simulink model "wlan_phy_rx_pmd"  (Simulink 7.8)
settings: Solver=VariableStepDiscrete  SolverMode=SingleTasking  StartTime=0.0  StopTime=rx_sim_time  RelTol=1e-3
PostLoadFcn:
  | wlan_phy_rx_init
InitFcn:
  | wlan_phy_rx_init
StartFcn:
  | wlan_phy_rx_init
values from neighbouring files (symbols this model uses):
  barker_seq20 = 0.95 * circshift(barker_seq20, [0 4]) ./ max(abs(barker_seq20))    [wlan_phy_rx_init.m]
  bit_scrambler_lfsr_bytes = sum(bit_scrambler_lfsr_bytes .* repmat(2.^[0:7], 127, 1),2)    [wlan_phy_rx_init.m]
  deinterleave_ROM = [deinterleave_ROM interleave_11n_64QAM zeros(1, 512-length(interleave_11n_64QAM))]    [wlan_phy_rx_init.m]
  longCorr_coef_i = [3*real(longCorr_coef)]    [wlan_phy_rx_init.m]
  longCorr_coef_q = [3*imag(longCorr_coef)]    [wlan_phy_rx_init.m]
  MAX_CP_LEN = 32    [wlan_phy_rx_init.m]
  MAX_NCBPS = NCBPS_11n_64QAM    (= 312)    [wlan_phy_rx_init.m]
  MAX_NUM_BYTES = 2^16-1    (= 65535)    [wlan_phy_rx_init.m]
  MAX_NUM_SC = 64    [wlan_phy_rx_init.m]
  MAX_NUM_SYMS = 600    [wlan_phy_rx_init.m]
  NCBPS_11a_BPSK = 48    [wlan_phy_rx_init.m]
  NDBPS_11a_BPSK12 = 24    [wlan_phy_rx_init.m]
  PHY_SIGNAL_MIN_LEN = 14    [wlan_phy_rx_init.m]
  preFFT_sampBuff_numSamps = 4*MAX_NUM_SC    (= 256)    [wlan_phy_rx_init.m]
  rx_sim_sig_samp_time = 8    [wlan_phy_rx_init.m]
  scr_ind_rev = zeros(1,128)    [wlan_phy_rx_init.m]
undefined symbols (not defined in the file or in its folder): gcbh, mcs_rom_11ag, mcs_rom_11n, xlBlockLoadCallback, xlGetNormalizedPeriod, xlipgetpartsetting
libraries: xbsIndex_r4 x3862, simulink x1, xbsEDKLib_r4 x1
(part 6 of 23)

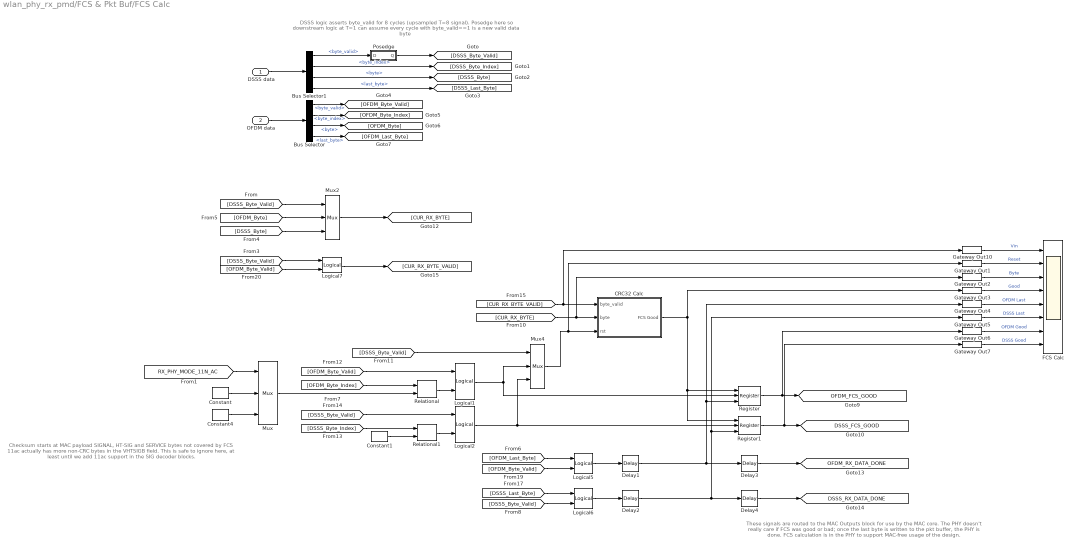
== system wlan_phy_rx_pmd/FCS & Pkt Buf/FCS Calc ==
  note: These signals are routed to the MAC Outputs
      | block for use by the MAC core. The PHY doesn't
      | really care if FCS was good or bad; once the
      | last byte is written to the pkt buffer, the PHY is done.
      | FCS calculation is in the PHY to support MAC-free
      | usage of the design.
  note: DSSS logic asserts byte_valid for 8 cycles (upsampled T=8 signal).
      | Posedge here so downstream logic at T=1 can assume every cycle
      | with byte_valid==1 is a new valid data byte
  note: Checksum starts at MAC payload
      | SIGNAL, HT-SIG and SERVICE
      | bytes not covered by FCS
      |
      | 11ac actually has more non-CRC bytes
      | in the VHTSIGB field. This is safe to
      | ignore here, at least until we add 11ac
      | support in the SIG decoder blocks.
  DSSS data : Inport
  OFDM data : Inport  Port=2
  Bus Selector : BusSelector  OutputSignals=byte_valid,byte_index,byte,last_byte
  Bus Selector1 : BusSelector  OutputSignals=byte_valid,byte_index,byte,last_byte
  CRC32 Calc : Subsystem
  Constant : xbsIndex_r4/Constant  const=4  gui_display_data_type=Fixed-point  arith_type=Unsigned  n_bits=3  bin_pt=0  preci_type=Single  exp_width=8  frac_width=24  explicit_period=off  period=1  equ=P=C  opselect=C  inp2=PCIN>>17  opr=+  inp1=P  carry=CIN  dbl_ovrd=off  block_type=constant
  Constant1 : xbsIndex_r4/Constant  const=5  gui_display_data_type=Fixed-point  arith_type=Unsigned  n_bits=3  bin_pt=0  preci_type=Single  exp_width=8  frac_width=24  explicit_period=off  period=1  equ=P=C  opselect=C  inp2=PCIN>>17  opr=+  inp1=P  carry=CIN  dbl_ovrd=off  block_type=constant
  Constant4 : xbsIndex_r4/Constant  const=10  gui_display_data_type=Fixed-point  arith_type=Unsigned  n_bits=4  bin_pt=0  preci_type=Single  exp_width=8  frac_width=24  explicit_period=off  period=1  equ=P=C  opselect=C  inp2=PCIN>>17  opr=+  inp1=P  carry=CIN  dbl_ovrd=off  block_type=constant
  Delay{1..4} (4 alike) : xbsIndex_r4/Delay  rst=off  infoeditControl=Selection of Reset will increase slice count due to use of real FFs and instead of SRLs  en=off  latency=1  dbl_ovrd=off  reg_retiming=off  block_type=delay
  FCS Calc : Scope  NumInputPorts=8
      settings: TimeRange=16000  YMin=0~0~0~-1~0~-1~-1~-1  YMax=1~1~275~1~1~1~1~1  SaveName=ScopeData22
  Gateway Out{1..7,10} (8 alike) : xbsIndex_r4/Gateway Out  inherit_from_input=off  hdl_port=off  timing_constraint=None  locs_specified=off  LOCs={}  UseAsDAC=off  DACChannel='1'  block_type=gatewayout
  Logical{1..2,5..6} (4 alike) : xbsIndex_r4/Logical  logical_function=AND  inputs=2  en=off  latency=0  precision=Full  arith_type=Unsigned  n_bits=8  bin_pt=2  align_bp=on  dbl_ovrd=off  block_type=logical
      settings: xl_area=[0, 0, 0, 0, 0, 0, 0]  block_version=VER_STRING_GOES_HERE
  Logical7 : xbsIndex_r4/Logical  logical_function=OR  inputs=2  en=off  latency=0  precision=Full  arith_type=Unsigned  n_bits=8  bin_pt=2  align_bp=on  dbl_ovrd=off  block_type=logical
      settings: xl_area=[0, 0, 0, 0, 0, 0, 0]  block_version=VER_STRING_GOES_HERE
  Mux : xbsIndex_r4/Mux  inputs=2  en=off  latency=0  precision=Full  arith_type=Unsigned  n_bits=16  bin_pt=14  quantization=Truncate  overflow=Wrap  dbl_ovrd=off  block_type=mux
  Mux2 : xbsIndex_r4/Mux  inputs=2  en=off  latency=0  precision=Full  arith_type=Unsigned  n_bits=16  bin_pt=14  quantization=Truncate  overflow=Wrap  dbl_ovrd=off  block_type=mux
  Mux4 : xbsIndex_r4/Mux  inputs=2  en=off  latency=0  precision=Full  arith_type=Unsigned  n_bits=16  bin_pt=14  quantization=Truncate  overflow=Wrap  dbl_ovrd=off  block_type=mux
  Posedge : Subsystem
  Register : xbsIndex_r4/Register  init=0  rst=on  en=on  dbl_ovrd=off  block_type=register
  Register1 : xbsIndex_r4/Register  init=0  rst=on  en=on  dbl_ovrd=off  block_type=register
  Relational : xbsIndex_r4/Relational  mode=a<=b  en=off  latency=0  dbl_ovrd=off  block_type=relational
  Relational1 : xbsIndex_r4/Relational  mode=a<=b  en=off  latency=0  dbl_ovrd=off  block_type=relational
  tag scope: DSSS_FCS_GOOD=global, DSSS_RX_DATA_DONE=global, OFDM_FCS_GOOD=global, OFDM_RX_DATA_DONE=global
  wires:
  [DSSS_Byte_Valid] -> Mux2/1
  [OFDM_Byte] -> Mux2/2
  [DSSS_Byte] -> Mux2/3
  Mux2/1 -> [CUR_RX_BYTE]
  [DSSS_Byte_Valid] -> Logical7/1
  [OFDM_Byte_Valid] -> Logical7/2
  Logical7/1 -> [CUR_RX_BYTE_VALID]
  Mux4/1 -> CRC32 Calc/3(rst), Gateway Out1/1
  [DSSS_Byte_Valid] -> Mux4/1
  Register1/1 -> [DSSS_FCS_GOOD], Gateway Out7/1
  Register/1 -> [OFDM_FCS_GOOD], Gateway Out6/1
  CRC32 Calc/1(FCS Good) -> Register/1, Register1/1, Gateway Out3/1
  Delay2/1 -> Delay4/1, Register1/3, Gateway Out5/1
  Delay1/1 -> Delay3/1, Register/3, Gateway Out4/1
  Logical6/1 -> Delay2/1
  Logical5/1 -> Delay1/1
  [DSSS_Byte_Valid] -> Logical6/2
  [DSSS_Last_Byte] -> Logical6/1
  [OFDM_Byte_Valid] -> Logical5/2
  [OFDM_Last_Byte] -> Logical5/1
  [CUR_RX_BYTE_VALID] -> CRC32 Calc/1(byte_valid), Gateway Out10/1
  Logical2/1 -> Mux4/3, Register1/2
  [DSSS_Byte_Valid] -> Logical2/1
  Relational1/1 -> Logical2/2
  Constant1/1 -> Relational1/2
  [DSSS_Byte_Index] -> Relational1/1
  Logical1/1 -> Mux4/2, Register/2
  [OFDM_Byte_Valid] -> Logical1/1
  Relational/1 -> Logical1/2
  Mux/1 -> Relational/2
  [OFDM_Byte_Index] -> Relational/1
  [CUR_RX_BYTE] -> CRC32 Calc/2(byte), Gateway Out2/1
  Delay3/1 -> [OFDM_RX_DATA_DONE]
  Delay4/1 -> [DSSS_RX_DATA_DONE]
  Gateway Out10/1 -> FCS Calc/1  "Vin"
  Gateway Out1/1 -> FCS Calc/2  "Reset"
  Gateway Out2/1 -> FCS Calc/3  "Byte"
  Gateway Out3/1 -> FCS Calc/4  "Good"
  Gateway Out4/1 -> FCS Calc/5  "OFDM Last"
  Gateway Out5/1 -> FCS Calc/6  "DSSS Last"
  Gateway Out6/1 -> FCS Calc/7  "OFDM Good"
  Gateway Out7/1 -> FCS Calc/8  "DSSS Good"
  Bus Selector1/1 -> Posedge/1(D)  "<byte_valid>"
  Bus Selector1/2 -> [DSSS_Byte_Index]  "<byte_index>"
  Bus Selector1/3 -> [DSSS_Byte]  "<byte>"
  Bus Selector1/4 -> [DSSS_Last_Byte]  "<last_byte>"
  Bus Selector/1 -> [OFDM_Byte_Valid]  "<byte_valid>"
  Bus Selector/2 -> [OFDM_Byte_Index]  "<byte_index>"
  Bus Selector/3 -> [OFDM_Byte]  "<byte>"
  Bus Selector/4 -> [OFDM_Last_Byte]  "<last_byte>"
  DSSS data/1 -> Bus Selector1/1
  OFDM data/1 -> Bus Selector/1
  Posedge/1(Q) -> [DSSS_Byte_Valid]
  [RX_PHY_MODE_11N_AC] -> Mux/1
  Constant/1 -> Mux/2
  Constant4/1 -> Mux/3

== system wlan_phy_rx_pmd/FCS & Pkt Buf/Pkt Buf Interface ==
  DSSS data : Inport
  OFDM data : Inport  Port=2
  Addr Check : Subsystem
  BRAM IF 64b : Subsystem
  Bus Selector : BusSelector  OutputSignals=byte_valid,byte_index,byte,last_byte
  Bus Selector1 : BusSelector  OutputSignals=byte_valid,byte_index,byte,last_byte
  DSSS Addr Calc : Subsystem
  Logical7 : xbsIndex_r4/Logical  logical_function=OR  inputs=2  en=off  latency=0  precision=Full  arith_type=Unsigned  n_bits=8  bin_pt=2  align_bp=on  dbl_ovrd=off  block_type=logical
      settings: xl_area=[0, 0, 0, 0, 0, 0, 0]  block_version=VER_STRING_GOES_HERE
  Mux2 : xbsIndex_r4/Mux  inputs=2  en=off  latency=0  precision=Full  arith_type=Unsigned  n_bits=16  bin_pt=14  quantization=Truncate  overflow=Wrap  dbl_ovrd=off  block_type=mux
  Mux3 : xbsIndex_r4/Mux  inputs=2  en=off  latency=0  precision=Full  arith_type=Unsigned  n_bits=16  bin_pt=14  quantization=Truncate  overflow=Wrap  dbl_ovrd=off  block_type=mux
  Mux5 : xbsIndex_r4/Mux  inputs=2  en=off  latency=0  precision=Full  arith_type=Unsigned  n_bits=16  bin_pt=14  quantization=Truncate  overflow=Wrap  dbl_ovrd=off  block_type=mux
  OFDM Addr Calc : Subsystem
  Register{4..5,7..8} (4 alike) : xbsIndex_r4/Register  init=0  rst=off  en=off  dbl_ovrd=off  block_type=register
  Rx Data MAC Outputs : Subsystem
  wires:
  [DSSS_Byte_Valid] -> Mux3/1
  [DSSS_Byte_Index] -> DSSS Addr Calc/1(R)
  DSSS Addr Calc/1(M) -> Mux5/3
  [DSSS_Byte_Valid] -> Mux5/1
  Mux5/1 -> Addr Check/2(Addr)
  [OFDM_Byte_Index] -> OFDM Addr Calc/1(R)
  OFDM Addr Calc/1(M) -> Mux5/2
  Mux3/1 -> Register4/1
  Register4/1 -> BRAM IF 64b/4(Pkt Buf Sel)
  [CUR_RX_BYTE] -> Register7/1
  [CUR_RX_BYTE_VALID] -> Addr Check/1(WrEn)
  Register7/1 -> BRAM IF 64b/1("byte "), Rx Data MAC Outputs/1("Byte ")
  Register8/1 -> BRAM IF 64b/2(byte_valid), Rx Data MAC Outputs/2(Byte Valid)
  Register5/1 -> BRAM IF 64b/3(byte_ind), Rx Data MAC Outputs/3(Byte Ind)
  Bus Selector1/1 -> [DSSS_Byte_Valid]  "<byte_valid>"
  Bus Selector1/2 -> [DSSS_Byte_Index]  "<byte_index>"
  Bus Selector1/3 -> [DSSS_Byte]  "<byte>"
  Bus Selector1/4 -> [DSSS_Last_Byte]  "<last_byte>"
  Bus Selector/1 -> [OFDM_Byte_Valid]  "<byte_valid>"
  Bus Selector/2 -> [OFDM_Byte_Index]  "<byte_index>"
  Bus Selector/3 -> [OFDM_Byte]  "<byte>"
  Bus Selector/4 -> [OFDM_Last_Byte]  "<last_byte>"
  DSSS data/1 -> Bus Selector1/1
  OFDM data/1 -> Bus Selector/1
  [DSSS_Byte_Valid] -> Mux2/1
  [OFDM_Byte] -> Mux2/2
  [DSSS_Byte] -> Mux2/3
  Mux2/1 -> [CUR_RX_BYTE]
  [DSSS_Byte_Valid] -> Logical7/1
  [OFDM_Byte_Valid] -> Logical7/2
  Logical7/1 -> [CUR_RX_BYTE_VALID]
  [regRx_OFDM_PKT_BUF_SEL] -> Mux3/2
  [regRx_DSSS_PKT_BUF_SEL] -> Mux3/3
  Addr Check/1(" WrEn") -> Register8/1
  Addr Check/2(" Addr") -> Register5/1

== system wlan_phy_rx_pmd/FFT/Reset Gen ==
  note: Extend FFT reset for duration of TVALID + 4 to handle
      | case of pkt ending while new FFT frame is being loaded.
      | Turns out reset inputs for FIFO and FFT do not actually
      | flush internal state - both cores keep respnding to inputs
      | while reset is asserted (very frustrating...)
  FFT Valid In : Inport
  Reset : Inport  Port=2
  Inverter : xbsIndex_r4/Inverter  en=off  latency=0  dbl_ovrd=off  block_type=inv
  Inverter1 : xbsIndex_r4/Inverter  en=off  latency=4  dbl_ovrd=off  block_type=inv
  Logical : xbsIndex_r4/Logical  logical_function=AND  inputs=2  en=off  latency=0  precision=Full  arith_type=Unsigned  n_bits=16  bin_pt=0  align_bp=on  dbl_ovrd=off  block_type=logical
  Logical1 : xbsIndex_r4/Logical  logical_function=OR  inputs=2  en=off  latency=0  precision=Full  arith_type=Unsigned  n_bits=16  bin_pt=0  align_bp=on  dbl_ovrd=off  block_type=logical
  Register2 : xbsIndex_r4/Register  init=1  rst=off  en=off  dbl_ovrd=off  block_type=register
      settings: xl_area=[1 1 0 0 0 0 0]  block_version=VER_STRING_GOES_HERE
  S-R Latch1 : Subsystem
  FFT Reset : Outport
  " Reset" : Outport  Port=2
  wires:
  Inverter1/1 -> S-R Latch1/2(R)
  Reset/1 -> Logical1/1, Logical/2
  Logical/1 -> S-R Latch1/1(S)
  S-R Latch1/1(Q) -> Logical1/2
  Logical1/1 -> " Reset"/1, Inverter/1
  FFT Valid In/1 -> Inverter1/1, Logical/1
  Inverter/1 -> Register2/1
  Register2/1 -> FFT Reset/1

== system wlan_phy_rx_pmd/FFT/Sym Count ==
  note: "Symbol index" counts OFDM symbols and increments with each FFT.
      | Content of each OFDM sybmol depends on PHY mode (ag, n, or ac)
  data_in_tvalid : Inport
  pkt_done : Inport  Port=2
  data_out_tlast : Inport  Port=3
  Logical : xbsIndex_r4/Logical  logical_function=AND  inputs=2  en=off  latency=0  precision=Full  arith_type=Unsigned  n_bits=16  bin_pt=0  align_bp=on  dbl_ovrd=off  block_type=logical
  S-R Latch1 : Subsystem
  Symbol Counter : xbsIndex_r4/Counter  cnt_type=Free Running  cnt_to=Inf  operation=Up  start_count=0  cnt_by_val=1  arith_type=Unsigned  n_bits=ceil(log2(MAX_NUM_SYMS))  bin_pt=0  load_pin=off  rst=on  en=on  explicit_period=off  period=1  dbl_ovrd=off  use_behavioral_HDL=off  implementation=Fabric  block_type=counter
  Sym Ind : Outport
  wires:
  data_out_tlast/1 -> Logical/1
  Logical/1 -> Symbol Counter/2
  S-R Latch1/1(Q) -> Logical/2
  data_in_tvalid/1 -> S-R Latch1/1(S)
  Symbol Counter/1 -> Sym Ind/1
  pkt_done/1 -> S-R Latch1/2(R), Symbol Counter/1

== system wlan_phy_rx_pmd/Inputs/RF A Inputs ==
  Rst : Inport
  IQ Valid : Inport  Port=2
  Constant1 : Constant  Value=0
  Constant2 : Constant  Value=2
  Constant4 : Constant  Value=5
  Delay1 : xbsIndex_r4/Delay  rst=off  infoeditControl=Selection of Reset will increase slice count due to use of real FFs and instead of SRLs  en=off  latency=1  dbl_ovrd=off  reg_retiming=off  block_type=delay
  Delay2 : xbsIndex_r4/Delay  rst=off  infoeditControl=Selection of Reset will increase slice count due to use of real FFs and instead of SRLs  en=off  latency=1  dbl_ovrd=off  reg_retiming=off  block_type=delay
  Down Sample : xbsIndex_r4/Down Sample  sample_ratio=16  sample_phase="Last Value of Frame  (most efficient)"  en=off  latency=1  dbl_ovrd=off  block_type=dsamp
  From Workspace : FromWorkspace  VariableName=rx_sim_sig_adc_I  SampleTime=rx_sim_sig_samp_time  Interpolate=off  OutputAfterFinalValue=Setting to zero
  From Workspace1 : FromWorkspace  VariableName=rx_sim_sig_adc_Q  SampleTime=rx_sim_sig_samp_time  Interpolate=off  OutputAfterFinalValue=Setting to zero
  RF A RSSI Sum : Subsystem
  RFA_G_BB : xbsIndex_r4/Gateway In  gui_display_data_type=Fixed-point  arith_type=Unsigned  n_bits=5  bin_pt=0  preci_type=Single  exp_width=8  frac_width=24  quantization="Round  (unbiased: +/- Inf)"  overflow=Saturate  period=1  dbl_ovrd=off  timing_constraint=None  locs_specified=off  LOCs={}  UseAsADC=off  ADCChannel='1'  inherit_from_input=off  hdl_port=on  block_type=gatewayin
  RFA_G_RF : xbsIndex_r4/Gateway In  gui_display_data_type=Fixed-point  arith_type=Unsigned  n_bits=2  bin_pt=0  preci_type=Single  exp_width=8  frac_width=24  quantization="Round  (unbiased: +/- Inf)"  overflow=Saturate  period=1  dbl_ovrd=off  timing_constraint=None  locs_specified=off  LOCs={}  UseAsADC=off  ADCChannel='1'  inherit_from_input=off  hdl_port=on  block_type=gatewayin
  RFA_RSSI : xbsIndex_r4/Gateway In  gui_display_data_type=Fixed-point  arith_type=Unsigned  n_bits=10  bin_pt=0  preci_type=Single  exp_width=8  frac_width=24  quantization="Round  (unbiased: +/- Inf)"  overflow=Saturate  period=1  dbl_ovrd=off  timing_constraint=None  locs_specified=off  LOCs={}  UseAsADC=off  ADCChannel='1'  inherit_from_input=off  hdl_port=on  block_type=gatewayin
  RFA_RX_I : xbsIndex_r4/Gateway In  gui_display_data_type=Fixed-point  arith_type="Signed  (2's comp)"  n_bits=16  bin_pt=15  preci_type=Single  exp_width=8  frac_width=24  quantization="Round  (unbiased: +/- Inf)"  overflow=Saturate  period=1  dbl_ovrd=off  timing_constraint=None  locs_specified=off  LOCs={}  UseAsADC=off  ADCChannel='1'  inherit_from_input=off  hdl_port=on  block_type=gatewayin
  RFA_RX_Q : xbsIndex_r4/Gateway In  gui_display_data_type=Fixed-point  arith_type="Signed  (2's comp)"  n_bits=16  bin_pt=15  preci_type=Single  exp_width=8  frac_width=24  quantization="Round  (unbiased: +/- Inf)"  overflow=Saturate  period=1  dbl_ovrd=off  timing_constraint=None  locs_specified=off  LOCs={}  UseAsADC=off  ADCChannel='1'  inherit_from_input=off  hdl_port=on  block_type=gatewayin
  Register : xbsIndex_r4/Register  init=0  rst=on  en=on  dbl_ovrd=off  block_type=register
  Register1 : xbsIndex_r4/Register  init=0  rst=on  en=on  dbl_ovrd=off  block_type=register
  Register2 : xbsIndex_r4/Register  init=0  rst=off  en=off  dbl_ovrd=off  block_type=register
  Register3 : xbsIndex_r4/Register  init=0  rst=on  en=off  dbl_ovrd=off  block_type=register
  Register4 : xbsIndex_r4/Register  init=0  rst=off  en=off  dbl_ovrd=off  block_type=register
  Register5 : xbsIndex_r4/Register  init=0  rst=off  en=off  dbl_ovrd=off  block_type=register
  ADC I : Outport
  ADC Q : Outport  Port=2
  Avg RSSI : Outport  Port=3
  tag scope: CS_ADC_I=global, CS_ADC_Q=global, CS_G_BB=global, CS_G_RF=global, CS_RFA_RSSI=global, RFA_AGC_G_BB=global, RFA_AGC_G_RF=global
  wires:
  From Workspace/1 -> RFA_RX_I/1
  From Workspace1/1 -> RFA_RX_Q/1
  RFA_RX_I/1 -> Register2/1
  RFA_RX_Q/1 -> Register4/1
  Register/1 -> ADC I/1
  Register1/1 -> ADC Q/1
  Register3/1 -> Down Sample/1
  Down Sample/1 -> RF A RSSI Sum/1(RSSI)
  RF A RSSI Sum/1(Sum) -> [CS_RFA_RSSI], Avg RSSI/1
  RFA_RSSI/1 -> Register5/1
  Register2/1 -> Register/1, [CS_ADC_I]
  Register4/1 -> Register1/1, [CS_ADC_Q]
  Register5/1 -> Register3/1
  Rst/1 -> Register/2, Register1/2, Register3/2
  IQ Valid/1 -> Register1/3, Register/3
  RFA_G_RF/1 -> Delay1/1
  RFA_G_BB/1 -> Delay2/1
  Delay1/1 -> [CS_G_RF], [RFA_AGC_G_RF]
  Delay2/1 -> [CS_G_BB], [RFA_AGC_G_BB]
  Constant2/1 -> RFA_G_RF/1
  Constant4/1 -> RFA_G_BB/1
  Constant1/1 -> RFA_RSSI/1

== system wlan_phy_rx_pmd/Inputs/RF B Inputs ==
  Rst : Inport
  IQ Valid : Inport  Port=2
  Constant1 : Constant  Value=0
  Constant2 : Constant  Value=0
  Constant3 : Constant  Value=0
  Constant6 : Constant  Value=2
  Constant7 : Constant  Value=5
  Delay4 : xbsIndex_r4/Delay  rst=off  infoeditControl=Selection of Reset will increase slice count due to use of real FFs and instead of SRLs  en=off  latency=1  dbl_ovrd=off  reg_retiming=off  block_type=delay
  Delay5 : xbsIndex_r4/Delay  rst=off  infoeditControl=Selection of Reset will increase slice count due to use of real FFs and instead of SRLs  en=off  latency=1  dbl_ovrd=off  reg_retiming=off  block_type=delay
  Down Sample : xbsIndex_r4/Down Sample  sample_ratio=16  sample_phase="Last Value of Frame  (most efficient)"  en=off  latency=1  dbl_ovrd=off  block_type=dsamp
  RF B RSSI Sum : Subsystem
  RFB_G_BB : xbsIndex_r4/Gateway In  gui_display_data_type=Fixed-point  arith_type=Unsigned  n_bits=5  bin_pt=0  preci_type=Single  exp_width=8  frac_width=24  quantization="Round  (unbiased: +/- Inf)"  overflow=Saturate  period=1  dbl_ovrd=off  timing_constraint=None  locs_specified=off  LOCs={}  UseAsADC=off  ADCChannel='1'  inherit_from_input=off  hdl_port=on  block_type=gatewayin
  RFB_G_RF : xbsIndex_r4/Gateway In  gui_display_data_type=Fixed-point  arith_type=Unsigned  n_bits=2  bin_pt=0  preci_type=Single  exp_width=8  frac_width=24  quantization="Round  (unbiased: +/- Inf)"  overflow=Saturate  period=1  dbl_ovrd=off  timing_constraint=None  locs_specified=off  LOCs={}  UseAsADC=off  ADCChannel='1'  inherit_from_input=off  hdl_port=on  block_type=gatewayin
  RFB_RSSI : xbsIndex_r4/Gateway In  gui_display_data_type=Fixed-point  arith_type=Unsigned  n_bits=10  bin_pt=0  preci_type=Single  exp_width=8  frac_width=24  quantization="Round  (unbiased: +/- Inf)"  overflow=Saturate  period=1  dbl_ovrd=off  timing_constraint=None  locs_specified=off  LOCs={}  UseAsADC=off  ADCChannel='1'  inherit_from_input=off  hdl_port=on  block_type=gatewayin
  RFB_RX_I : xbsIndex_r4/Gateway In  gui_display_data_type=Fixed-point  arith_type="Signed  (2's comp)"  n_bits=16  bin_pt=15  preci_type=Single  exp_width=8  frac_width=24  quantization="Round  (unbiased: +/- Inf)"  overflow=Saturate  period=1  dbl_ovrd=off  timing_constraint=None  locs_specified=off  LOCs={}  UseAsADC=off  ADCChannel='1'  inherit_from_input=off  hdl_port=on  block_type=gatewayin
  RFB_RX_Q : xbsIndex_r4/Gateway In  gui_display_data_type=Fixed-point  arith_type="Signed  (2's comp)"  n_bits=16  bin_pt=15  preci_type=Single  exp_width=8  frac_width=24  quantization="Round  (unbiased: +/- Inf)"  overflow=Saturate  period=1  dbl_ovrd=off  timing_constraint=None  locs_specified=off  LOCs={}  UseAsADC=off  ADCChannel='1'  inherit_from_input=off  hdl_port=on  block_type=gatewayin
  Register : xbsIndex_r4/Register  init=0  rst=on  en=on  dbl_ovrd=off  block_type=register
  Register1 : xbsIndex_r4/Register  init=0  rst=on  en=on  dbl_ovrd=off  block_type=register
  Register2 : xbsIndex_r4/Register  init=0  rst=off  en=off  dbl_ovrd=off  block_type=register
  Register3 : xbsIndex_r4/Register  init=0  rst=on  en=off  dbl_ovrd=off  block_type=register
  Register4 : xbsIndex_r4/Register  init=0  rst=off  en=off  dbl_ovrd=off  block_type=register
  Register5 : xbsIndex_r4/Register  init=0  rst=off  en=off  dbl_ovrd=off  block_type=register
  ADC I : Outport
  ADC Q : Outport  Port=2
  Avg RSSI : Outport  Port=3
  tag scope: RFB_AGC_G_BB=global, RFB_AGC_G_RF=global
  wires:
  IQ Valid/1 -> Register/3, Register1/3
  Rst/1 -> Register3/2, Register1/2, Register/2
  Register5/1 -> Register3/1
  Register4/1 -> Register1/1
  Register2/1 -> Register/1
  RFB_RSSI/1 -> Register5/1
  RF B RSSI Sum/1(Sum) -> Avg RSSI/1
  Down Sample/1 -> RF B RSSI Sum/1(RSSI)
  Register3/1 -> Down Sample/1
  Register1/1 -> ADC Q/1
  Register/1 -> ADC I/1
  RFB_RX_Q/1 -> Register4/1
  RFB_RX_I/1 -> Register2/1
  RFB_G_RF/1 -> Delay4/1
  RFB_G_BB/1 -> Delay5/1
  Delay4/1 -> [RFB_AGC_G_RF]
  Delay5/1 -> [RFB_AGC_G_BB]
  Constant6/1 -> RFB_G_RF/1
  Constant7/1 -> RFB_G_BB/1
  Constant1/1 -> RFB_RX_I/1
  Constant3/1 -> RFB_RSSI/1
  Constant2/1 -> RFB_RX_Q/1

== system wlan_phy_rx_pmd/Inputs/RF C Inputs ==
  Rst : Inport
  IQ Valid : Inport  Port=2
  Constant1 : Constant  Value=0
  Constant2 : Constant  Value=2
  Constant3 : Constant  Value=0
  Constant4 : Constant  Value=5
  Constant5 : Constant  Value=0
  Delay1 : xbsIndex_r4/Delay  rst=off  infoeditControl=Selection of Reset will increase slice count due to use of real FFs and instead of SRLs  en=off  latency=1  dbl_ovrd=off  reg_retiming=off  block_type=delay
  Delay2 : xbsIndex_r4/Delay  rst=off  infoeditControl=Selection of Reset will increase slice count due to use of real FFs and instead of SRLs  en=off  latency=1  dbl_ovrd=off  reg_retiming=off  block_type=delay
  Down Sample : xbsIndex_r4/Down Sample  sample_ratio=16  sample_phase="Last Value of Frame  (most efficient)"  en=off  latency=1  dbl_ovrd=off  block_type=dsamp
  RF C RSSI Sum : Subsystem
  RFC_G_BB : xbsIndex_r4/Gateway In  gui_display_data_type=Fixed-point  arith_type=Unsigned  n_bits=5  bin_pt=0  preci_type=Single  exp_width=8  frac_width=24  quantization="Round  (unbiased: +/- Inf)"  overflow=Saturate  period=1  dbl_ovrd=off  timing_constraint=None  locs_specified=off  LOCs={}  UseAsADC=off  ADCChannel='1'  inherit_from_input=off  hdl_port=on  block_type=gatewayin
  RFC_G_RF : xbsIndex_r4/Gateway In  gui_display_data_type=Fixed-point  arith_type=Unsigned  n_bits=2  bin_pt=0  preci_type=Single  exp_width=8  frac_width=24  quantization="Round  (unbiased: +/- Inf)"  overflow=Saturate  period=1  dbl_ovrd=off  timing_constraint=None  locs_specified=off  LOCs={}  UseAsADC=off  ADCChannel='1'  inherit_from_input=off  hdl_port=on  block_type=gatewayin
  RFC_RSSI : xbsIndex_r4/Gateway In  gui_display_data_type=Fixed-point  arith_type=Unsigned  n_bits=10  bin_pt=0  preci_type=Single  exp_width=8  frac_width=24  quantization="Round  (unbiased: +/- Inf)"  overflow=Saturate  period=1  dbl_ovrd=off  timing_constraint=None  locs_specified=off  LOCs={}  UseAsADC=off  ADCChannel='1'  inherit_from_input=off  hdl_port=on  block_type=gatewayin
  RFC_RX_I : xbsIndex_r4/Gateway In  gui_display_data_type=Fixed-point  arith_type="Signed  (2's comp)"  n_bits=16  bin_pt=15  preci_type=Single  exp_width=8  frac_width=24  quantization="Round  (unbiased: +/- Inf)"  overflow=Saturate  period=1  dbl_ovrd=off  timing_constraint=None  locs_specified=off  LOCs={}  UseAsADC=off  ADCChannel='1'  inherit_from_input=off  hdl_port=on  block_type=gatewayin
  RFC_RX_Q : xbsIndex_r4/Gateway In  gui_display_data_type=Fixed-point  arith_type="Signed  (2's comp)"  n_bits=16  bin_pt=15  preci_type=Single  exp_width=8  frac_width=24  quantization="Round  (unbiased: +/- Inf)"  overflow=Saturate  period=1  dbl_ovrd=off  timing_constraint=None  locs_specified=off  LOCs={}  UseAsADC=off  ADCChannel='1'  inherit_from_input=off  hdl_port=on  block_type=gatewayin
  Register : xbsIndex_r4/Register  init=0  rst=on  en=on  dbl_ovrd=off  block_type=register
  Register1 : xbsIndex_r4/Register  init=0  rst=on  en=on  dbl_ovrd=off  block_type=register
  Register2 : xbsIndex_r4/Register  init=0  rst=off  en=off  dbl_ovrd=off  block_type=register
  Register3 : xbsIndex_r4/Register  init=0  rst=on  en=off  dbl_ovrd=off  block_type=register
  Register4 : xbsIndex_r4/Register  init=0  rst=off  en=off  dbl_ovrd=off  block_type=register
  Register5 : xbsIndex_r4/Register  init=0  rst=off  en=off  dbl_ovrd=off  block_type=register
  ADC I : Outport
  ADC Q : Outport  Port=2
  Avg RSSI : Outport  Port=3
  tag scope: CS_ADC_I_RFC=global, CS_ADC_Q_RFC=global, RFC_AGC_G_BB=global, RFC_AGC_G_RF=global
  wires:
  Constant4/1 -> RFC_G_BB/1
  Constant2/1 -> RFC_G_RF/1
  Delay2/1 -> [RFC_AGC_G_BB]
  Delay1/1 -> [RFC_AGC_G_RF]
  RFC_G_BB/1 -> Delay2/1
  RFC_G_RF/1 -> Delay1/1
  IQ Valid/1 -> Register/3, Register1/3
  Rst/1 -> Register3/2, Register1/2, Register/2
  Register5/1 -> Register3/1
  Register4/1 -> Register1/1, [CS_ADC_Q_RFC]
  Register2/1 -> Register/1, [CS_ADC_I_RFC]
  RFC_RSSI/1 -> Register5/1
  RF C RSSI Sum/1(Sum) -> Avg RSSI/1
  Down Sample/1 -> RF C RSSI Sum/1(RSSI)
  Register3/1 -> Down Sample/1
  Register1/1 -> ADC Q/1
  Register/1 -> ADC I/1
  RFC_RX_Q/1 -> Register4/1
  RFC_RX_I/1 -> Register2/1
  Constant3/1 -> RFC_RX_Q/1
  Constant1/1 -> RFC_RX_I/1
  Constant5/1 -> RFC_RSSI/1

== system wlan_phy_rx_pmd/Inputs/RF D Inputs ==
  Rst : Inport
  IQ Valid : Inport  Port=2
  Constant1 : Constant  Value=0
  Constant2 : Constant  Value=0
  Constant3 : Constant  Value=0
  Constant6 : Constant  Value=2
  Constant7 : Constant  Value=5
  Delay4 : xbsIndex_r4/Delay  rst=off  infoeditControl=Selection of Reset will increase slice count due to use of real FFs and instead of SRLs  en=off  latency=1  dbl_ovrd=off  reg_retiming=off  block_type=delay
  Delay5 : xbsIndex_r4/Delay  rst=off  infoeditControl=Selection of Reset will increase slice count due to use of real FFs and instead of SRLs  en=off  latency=1  dbl_ovrd=off  reg_retiming=off  block_type=delay
  Down Sample : xbsIndex_r4/Down Sample  sample_ratio=16  sample_phase="Last Value of Frame  (most efficient)"  en=off  latency=1  dbl_ovrd=off  block_type=dsamp
  RF D RSSI Sum : Subsystem
  RFD_G_BB : xbsIndex_r4/Gateway In  gui_display_data_type=Fixed-point  arith_type=Unsigned  n_bits=5  bin_pt=0  preci_type=Single  exp_width=8  frac_width=24  quantization="Round  (unbiased: +/- Inf)"  overflow=Saturate  period=1  dbl_ovrd=off  timing_constraint=None  locs_specified=off  LOCs={}  UseAsADC=off  ADCChannel='1'  inherit_from_input=off  hdl_port=on  block_type=gatewayin
  RFD_G_RF : xbsIndex_r4/Gateway In  gui_display_data_type=Fixed-point  arith_type=Unsigned  n_bits=2  bin_pt=0  preci_type=Single  exp_width=8  frac_width=24  quantization="Round  (unbiased: +/- Inf)"  overflow=Saturate  period=1  dbl_ovrd=off  timing_constraint=None  locs_specified=off  LOCs={}  UseAsADC=off  ADCChannel='1'  inherit_from_input=off  hdl_port=on  block_type=gatewayin
  RFD_RSSI : xbsIndex_r4/Gateway In  gui_display_data_type=Fixed-point  arith_type=Unsigned  n_bits=10  bin_pt=0  preci_type=Single  exp_width=8  frac_width=24  quantization="Round  (unbiased: +/- Inf)"  overflow=Saturate  period=1  dbl_ovrd=off  timing_constraint=None  locs_specified=off  LOCs={}  UseAsADC=off  ADCChannel='1'  inherit_from_input=off  hdl_port=on  block_type=gatewayin
  RFD_RX_I : xbsIndex_r4/Gateway In  gui_display_data_type=Fixed-point  arith_type="Signed  (2's comp)"  n_bits=16  bin_pt=15  preci_type=Single  exp_width=8  frac_width=24  quantization="Round  (unbiased: +/- Inf)"  overflow=Saturate  period=1  dbl_ovrd=off  timing_constraint=None  locs_specified=off  LOCs={}  UseAsADC=off  ADCChannel='1'  inherit_from_input=off  hdl_port=on  block_type=gatewayin
  RFD_RX_Q : xbsIndex_r4/Gateway In  gui_display_data_type=Fixed-point  arith_type="Signed  (2's comp)"  n_bits=16  bin_pt=15  preci_type=Single  exp_width=8  frac_width=24  quantization="Round  (unbiased: +/- Inf)"  overflow=Saturate  period=1  dbl_ovrd=off  timing_constraint=None  locs_specified=off  LOCs={}  UseAsADC=off  ADCChannel='1'  inherit_from_input=off  hdl_port=on  block_type=gatewayin
  Register : xbsIndex_r4/Register  init=0  rst=on  en=on  dbl_ovrd=off  block_type=register
  Register1 : xbsIndex_r4/Register  init=0  rst=on  en=on  dbl_ovrd=off  block_type=register
  Register2 : xbsIndex_r4/Register  init=0  rst=off  en=off  dbl_ovrd=off  block_type=register
  Register3 : xbsIndex_r4/Register  init=0  rst=on  en=off  dbl_ovrd=off  block_type=register
  Register4 : xbsIndex_r4/Register  init=0  rst=off  en=off  dbl_ovrd=off  block_type=register
  Register5 : xbsIndex_r4/Register  init=0  rst=off  en=off  dbl_ovrd=off  block_type=register
  ADC I : Outport
  ADC Q : Outport  Port=2
  Avg RSSI : Outport  Port=3
  tag scope: RFD_AGC_G_BB=global, RFD_AGC_G_RF=global
  wires:
  Constant7/1 -> RFD_G_BB/1
  Constant6/1 -> RFD_G_RF/1
  Delay5/1 -> [RFD_AGC_G_BB]
  Delay4/1 -> [RFD_AGC_G_RF]
  RFD_G_BB/1 -> Delay5/1
  RFD_G_RF/1 -> Delay4/1
  RFD_RX_I/1 -> Register2/1
  RFD_RX_Q/1 -> Register4/1
  Register/1 -> ADC I/1
  Register1/1 -> ADC Q/1
  Register3/1 -> Down Sample/1
  Down Sample/1 -> RF D RSSI Sum/1(RSSI)
  RF D RSSI Sum/1(Sum) -> Avg RSSI/1
  RFD_RSSI/1 -> Register5/1
  Register2/1 -> Register/1
  Register4/1 -> Register1/1
  Register5/1 -> Register3/1
  Rst/1 -> Register/2, Register1/2, Register3/2
  IQ Valid/1 -> Register1/3, Register/3
  Constant1/1 -> RFD_RX_I/1
  Constant2/1 -> RFD_RX_Q/1
  Constant3/1 -> RFD_RSSI/1

== system wlan_phy_rx_pmd/Inputs/RSSI Clock Gen ==
  1LSB+3 : xbsIndex_r4/Slice  nbits=1  boolean_output=off  mode=Lower Bit Location + Width  bit1=0  base1=MSB of Input  bit0=3  base0=LSB of Input  dbl_ovrd=off  block_type=slice
  RSSI Clock Generator : xbsIndex_r4/Counter  cnt_type=Free Running  cnt_to=Inf  operation=Up  start_count=0  cnt_by_val=1  arith_type=Unsigned  n_bits=4  bin_pt=0  load_pin=off  rst=off  en=off  explicit_period=on  period=1  dbl_ovrd=off  use_behavioral_HDL=off  implementation=Fabric  use_rpm=off  block_type=counter
      settings: block_version=VER_STRING_GOES_HERE
  Clk : Outport
  wires:
  RSSI Clock Generator/1 -> 1LSB+3/1
  1LSB+3/1 -> Clk/1

== system wlan_phy_rx_pmd/Inputs/negedge1 ==
  D : Inport
  Delay : xbsIndex_r4/Delay  rst=off  infoeditControl=Selection of Reset will increase slice count due to use of real FFs and instead of SRLs  en=off  latency=1  dbl_ovrd=off  reg_retiming=off  block_type=delay
      settings: infoedit=Hardware notes: A delay line is a chain, each link of which is an SRL16 followed by a flip-flop. If register retiming is enabled, the delay line is a chain of flip-flops.  xl_area=[1 1 0 0 0 0 0]  block_version=VER_STRING_GOES_HERE
  Inverter : xbsIndex_r4/Inverter  en=off  latency=0  dbl_ovrd=off  block_type=inv
      settings: xl_area=[0 0 0 0 0 0 0]  block_version=VER_STRING_GOES_HERE
  Logical1 : xbsIndex_r4/Logical  logical_function=AND  inputs=2  en=off  latency=0  precision=Full  arith_type=Unsigned  n_bits=8  bin_pt=2  align_bp=on  dbl_ovrd=off  block_type=logical
      settings: xl_area=[1 0 0 1 0 0 0]  block_version=VER_STRING_GOES_HERE
  Q : Outport
  wires:
  D/1 -> Delay/1, Inverter/1
  Logical1/1 -> Q/1
  Delay/1 -> Logical1/1
  Inverter/1 -> Logical1/2

== system wlan_phy_rx_pmd/MAC Outputs/Pulse Stretch1 ==
  P : Inport
  Delay : xbsIndex_r4/Delay  rst=off  infoeditControl=Selection of Reset will increase slice count due to use of real FFs and instead of SRLs  en=off  latency=16  dbl_ovrd=off  reg_retiming=off  block_type=delay
  S-R Latch : Subsystem
  P16 : Outport
  wires:
  S-R Latch/1(Q) -> Delay/1, P16/1
  Delay/1 -> S-R Latch/2(R)
  P/1 -> S-R Latch/1(S)

== system wlan_phy_rx_pmd/MAC Outputs/S-R Latch1 ==
  S : Inport
  R : Inport  Port=2
  Constant1 : xbsIndex_r4/Constant  const=1  gui_display_data_type=Fixed-point  arith_type=Boolean  n_bits=1  bin_pt=0  preci_type=Single  exp_width=8  frac_width=24  explicit_period=off  period=1  equ=P=C  opselect=C  inp2=PCIN>>17  opr=+  inp1=P  carry=CIN  dbl_ovrd=off  block_type=constant
      settings: block_version=VER_STRING_GOES_HERE
  Convert1 : xbsIndex_r4/Convert  gui_display_data_type=Boolean  arith_type=Boolean  n_bits=1  bin_pt=0  float_type=Single  exp_bits=8  fraction_bits=24  quantization=Truncate  overflow=Wrap  en=off  latency=0  dbl_ovrd=off  pipeline=off  block_type=convert
  Convert2 : xbsIndex_r4/Convert  gui_display_data_type=Boolean  arith_type=Boolean  n_bits=1  bin_pt=0  float_type=Single  exp_bits=8  fraction_bits=24  quantization=Truncate  overflow=Wrap  en=off  latency=0  dbl_ovrd=off  pipeline=off  block_type=convert
  Register2 : xbsIndex_r4/Register  init=0  rst=on  en=on  dbl_ovrd=off  block_type=register
      settings: xl_area=[1 1 0 0 0 0 0]  block_version=VER_STRING_GOES_HERE
  Q : Outport
  wires:
  Convert2/1 -> Register2/3
  Convert1/1 -> Register2/2
  Constant1/1 -> Register2/1
  R/1 -> Convert1/1
  Register2/1 -> Q/1
  S/1 -> Convert2/1

== system wlan_phy_rx_pmd/MAC Outputs/S-R Latch2 ==
  S : Inport
  R : Inport  Port=2
  Constant1 : xbsIndex_r4/Constant  const=1  gui_display_data_type=Fixed-point  arith_type=Boolean  n_bits=1  bin_pt=0  preci_type=Single  exp_width=8  frac_width=24  explicit_period=off  period=1  equ=P=C  opselect=C  inp2=PCIN>>17  opr=+  inp1=P  carry=CIN  dbl_ovrd=off  block_type=constant
      settings: block_version=VER_STRING_GOES_HERE
  Convert1 : xbsIndex_r4/Convert  gui_display_data_type=Boolean  arith_type=Boolean  n_bits=1  bin_pt=0  float_type=Single  exp_bits=8  fraction_bits=24  quantization=Truncate  overflow=Wrap  en=off  latency=0  dbl_ovrd=off  pipeline=off  block_type=convert
  Convert2 : xbsIndex_r4/Convert  gui_display_data_type=Boolean  arith_type=Boolean  n_bits=1  bin_pt=0  float_type=Single  exp_bits=8  fraction_bits=24  quantization=Truncate  overflow=Wrap  en=off  latency=0  dbl_ovrd=off  pipeline=off  block_type=convert
  Register2 : xbsIndex_r4/Register  init=0  rst=on  en=on  dbl_ovrd=off  block_type=register
      settings: xl_area=[1 1 0 0 0 0 0]  block_version=VER_STRING_GOES_HERE
  Q : Outport
  wires:
  S/1 -> Convert2/1
  Register2/1 -> Q/1
  R/1 -> Convert1/1
  Constant1/1 -> Register2/1
  Convert1/1 -> Register2/2
  Convert2/1 -> Register2/3

== system wlan_phy_rx_pmd/Registers/2x  ==
  d : Inport
  Concat2 : xbsIndex_r4/Concat  num_inputs=2  dbl_ovrd=off  block_type=concat
      settings: block_version=8.2.02
  Constant2 : xbsIndex_r4/Constant  const=0  gui_display_data_type=Fixed-point  arith_type=Unsigned  n_bits=1  bin_pt=0  preci_type=Single  exp_width=8  frac_width=24  explicit_period=on  period=1  equ=P=C  opselect=C  inp2=PCIN>>17  opr=+  inp1=P  carry=CIN  dbl_ovrd=off  block_type=constant
  2d : Outport
  wires:
  Concat2/1 -> 2d/1
  d/1 -> Concat2/1
  Constant2/1 -> Concat2/2

== system wlan_phy_rx_pmd/Registers/Config Bits ==
  32b : Inport
  "b[0]   " : xbsIndex_r4/Slice  nbits=1  boolean_output=on  mode=Lower Bit Location + Width  bit1=0  base1=MSB of Input  bit0=0  base0=LSB of Input  dbl_ovrd=off  block_type=slice
      settings: block_version=VER_STRING_GOES_HERE
  b[10] : xbsIndex_r4/Slice  nbits=1  boolean_output=on  mode=Lower Bit Location + Width  bit1=0  base1=MSB of Input  bit0=10  base0=LSB of Input  dbl_ovrd=off  block_type=slice
      settings: block_version=VER_STRING_GOES_HERE
  b[11] : xbsIndex_r4/Slice  nbits=1  boolean_output=on  mode=Lower Bit Location + Width  bit1=0  base1=MSB of Input  bit0=11  base0=LSB of Input  dbl_ovrd=off  block_type=slice
      settings: block_version=VER_STRING_GOES_HERE
  b[12] : xbsIndex_r4/Slice  nbits=1  boolean_output=on  mode=Lower Bit Location + Width  bit1=0  base1=MSB of Input  bit0=12  base0=LSB of Input  dbl_ovrd=off  block_type=slice
      settings: block_version=VER_STRING_GOES_HERE
  b[13] : xbsIndex_r4/Slice  nbits=1  boolean_output=on  mode=Lower Bit Location + Width  bit1=0  base1=MSB of Input  bit0=13  base0=LSB of Input  dbl_ovrd=off  block_type=slice
      settings: block_version=VER_STRING_GOES_HERE
  b[14] : xbsIndex_r4/Slice  nbits=1  boolean_output=on  mode=Lower Bit Location + Width  bit1=0  base1=MSB of Input  bit0=14  base0=LSB of Input  dbl_ovrd=off  block_type=slice
      settings: block_version=VER_STRING_GOES_HERE
  b[16:15] : xbsIndex_r4/Slice  nbits=2  boolean_output=off  mode=Lower Bit Location + Width  bit1=0  base1=MSB of Input  bit0=15  base0=LSB of Input  dbl_ovrd=off  block_type=slice
      settings: block_version=VER_STRING_GOES_HERE
  "b[1]  " : xbsIndex_r4/Slice  nbits=1  boolean_output=on  mode=Lower Bit Location + Width  bit1=0  base1=MSB of Input  bit0=1  base0=LSB of Input  dbl_ovrd=off  block_type=slice
      settings: block_version=VER_STRING_GOES_HERE
  b[20:17] : xbsIndex_r4/Slice  nbits=4  boolean_output=off  mode=Lower Bit Location + Width  bit1=0  base1=MSB of Input  bit0=17  base0=LSB of Input  dbl_ovrd=off  block_type=slice
      settings: block_version=VER_STRING_GOES_HERE
  b[21] : xbsIndex_r4/Slice  nbits=1  boolean_output=on  mode=Lower Bit Location + Width  bit1=0  base1=MSB of Input  bit0=21  base0=LSB of Input  dbl_ovrd=off  block_type=slice
      settings: block_version=VER_STRING_GOES_HERE
  b[22] : xbsIndex_r4/Slice  nbits=1  boolean_output=on  mode=Lower Bit Location + Width  bit1=0  base1=MSB of Input  bit0=22  base0=LSB of Input  dbl_ovrd=off  block_type=slice
      settings: block_version=VER_STRING_GOES_HERE
  b[23] : xbsIndex_r4/Slice  nbits=1  boolean_output=on  mode=Lower Bit Location + Width  bit1=0  base1=MSB of Input  bit0=23  base0=LSB of Input  dbl_ovrd=off  block_type=slice
      settings: block_version=VER_STRING_GOES_HERE
  b[2] : xbsIndex_r4/Slice  nbits=1  boolean_output=off  mode=Lower Bit Location + Width  bit1=0  base1=MSB of Input  bit0=2  base0=LSB of Input  dbl_ovrd=off  block_type=slice
      settings: block_version=VER_STRING_GOES_HERE
  b[3] : xbsIndex_r4/Slice  nbits=1  boolean_output=off  mode=Lower Bit Location + Width  bit1=0  base1=MSB of Input  bit0=3  base0=LSB of Input  dbl_ovrd=off  block_type=slice
      settings: block_version=VER_STRING_GOES_HERE
  b[4] : xbsIndex_r4/Slice  nbits=1  boolean_output=off  mode=Lower Bit Location + Width  bit1=0  base1=MSB of Input  bit0=4  base0=LSB of Input  dbl_ovrd=off  block_type=slice
      settings: block_version=VER_STRING_GOES_HERE
  b[5] : xbsIndex_r4/Slice  nbits=1  boolean_output=on  mode=Lower Bit Location + Width  bit1=0  base1=MSB of Input  bit0=5  base0=LSB of Input  dbl_ovrd=off  block_type=slice
      settings: block_version=VER_STRING_GOES_HERE
  b[6] : xbsIndex_r4/Slice  nbits=1  boolean_output=on  mode=Lower Bit Location + Width  bit1=0  base1=MSB of Input  bit0=6  base0=LSB of Input  dbl_ovrd=off  block_type=slice
      settings: block_version=VER_STRING_GOES_HERE
  b[7] : xbsIndex_r4/Slice  nbits=1  boolean_output=on  mode=Lower Bit Location + Width  bit1=0  base1=MSB of Input  bit0=7  base0=LSB of Input  dbl_ovrd=off  block_type=slice
      settings: block_version=VER_STRING_GOES_HERE
  b[8] : xbsIndex_r4/Slice  nbits=1  boolean_output=on  mode=Lower Bit Location + Width  bit1=0  base1=MSB of Input  bit0=8  base0=LSB of Input  dbl_ovrd=off  block_type=slice
      settings: block_version=VER_STRING_GOES_HERE
  b[9] : xbsIndex_r4/Slice  nbits=1  boolean_output=on  mode=Lower Bit Location + Width  bit1=0  base1=MSB of Input  bit0=9  base0=LSB of Input  dbl_ovrd=off  block_type=slice
      settings: block_version=VER_STRING_GOES_HERE
  tag scope: REG_USE_RX_SIGS_INVALID_INPUT=global, regRx_BYPASS_CFO_EST=global, regRx_DSSS_RX_EN=global, regRx_DSSS_RX_PKT_DET_OUT_EN=global, regRx_DSSS_RX_REQ_AGC_DONE=global, regRx_DSSS_asserts_CCA_busy=global, regRx_MAX_SIGNAL_LENGTH_KB=global, regRx_PKT_BUF_BYTE_ORDER_SWAP=global, regRx_PKT_BUF_H_EST_ORDER_SWAP=global, regRx_Record_Chan_Est_Pkt_Buf=global, regRx_noSwitching_AntSel=global, regRx_phyCCA_Mode_Sel=global, regRx_pktDetEn_Ext=global, regRx_pktDetEn_RFA=global, regRx_pktDetEn_RFB=global, regRx_pktDetEn_RFC=global, regRx_pktDetEn_RFD=global, regRx_pktDet_requireBoth=global, regRx_rateLength_holds_pktDet=global, regRx_switchingDiv_En=global
  wires:
  32b/1 -> "b[1]  "/1, b[2]/1, b[3]/1, b[4]/1, b[5]/1, b[6]/1, b[7]/1, b[9]/1, b[10]/1, b[12]/1, b[13]/1, b[14]/1, b[16:15]/1, b[20:17]/1, b[21]/1, b[22]/1, b[23]/1, b[11]/1, b[8]/1, "b[0]   "/1
  "b[0]   "/1 -> [regRx_DSSS_RX_EN]
  "b[1]  "/1 -> [REG_USE_RX_SIGS_INVALID_INPUT]
  b[2]/1 -> [regRx_PKT_BUF_BYTE_ORDER_SWAP]
  b[3]/1 -> [regRx_PKT_BUF_H_EST_ORDER_SWAP]
  b[4]/1 -> [regRx_DSSS_RX_PKT_DET_OUT_EN]
  b[5]/1 -> [regRx_BYPASS_CFO_EST]
  b[6]/1 -> [regRx_Record_Chan_Est_Pkt_Buf]
  b[7]/1 -> [regRx_switchingDiv_En]
  b[16:15]/1 -> [regRx_noSwitching_AntSel]
  b[9]/1 -> [regRx_pktDetEn_RFA]
  b[10]/1 -> [regRx_pktDetEn_RFB]
  b[13]/1 -> [regRx_pktDetEn_Ext]
  b[11]/1 -> [regRx_pktDetEn_RFC]
  b[12]/1 -> [regRx_pktDetEn_RFD]
  b[14]/1 -> [regRx_phyCCA_Mode_Sel]
  b[8]/1 -> [regRx_DSSS_RX_REQ_AGC_DONE]
  b[20:17]/1 -> [regRx_MAX_SIGNAL_LENGTH_KB]
  b[21]/1 -> [regRx_pktDet_requireBoth]
  b[22]/1 -> [regRx_rateLength_holds_pktDet]
  b[23]/1 -> [regRx_DSSS_asserts_CCA_busy]

== system wlan_phy_rx_pmd/Registers/Fanout ==
  Corr_Thresh : Inport
  E_Thresh : Inport  Port=2
  MinDur : Inport  Port=3
  Register{1..11,20} (12 alike) : xbsIndex_r4/Register  init=0  rst=off  en=off  dbl_ovrd=off  block_type=register
  tag scope: regPktDet_pktDetMinDuration_autoCorr_A=global, regPktDet_pktDetMinDuration_autoCorr_B=global, regPktDet_pktDetMinDuration_autoCorr_C=global, regPktDet_pktDetMinDuration_autoCorr_D=global, regRx_PktDet_CorrThresh_A=global, regRx_PktDet_CorrThresh_B=global, regRx_PktDet_CorrThresh_C=global, regRx_PktDet_CorrThresh_D=global, regRx_PktDet_EnergyThresh_A=global, regRx_PktDet_EnergyThresh_B=global, regRx_PktDet_EnergyThresh_C=global, regRx_PktDet_EnergyThresh_D=global
  wires:
  Corr_Thresh/1 -> Register20/1, Register1/1, Register2/1, Register3/1
  E_Thresh/1 -> Register4/1, Register5/1, Register6/1, Register7/1
  MinDur/1 -> Register8/1, Register9/1, Register10/1, Register11/1
  Register20/1 -> [regRx_PktDet_CorrThresh_D]
  Register1/1 -> [regRx_PktDet_CorrThresh_C]
  Register2/1 -> [regRx_PktDet_CorrThresh_B]
  Register3/1 -> [regRx_PktDet_CorrThresh_A]
  Register4/1 -> [regRx_PktDet_EnergyThresh_A]
  Register5/1 -> [regRx_PktDet_EnergyThresh_B]
  Register6/1 -> [regRx_PktDet_EnergyThresh_C]
  Register7/1 -> [regRx_PktDet_EnergyThresh_D]
  Register8/1 -> [regPktDet_pktDetMinDuration_autoCorr_A]
  Register9/1 -> [regPktDet_pktDetMinDuration_autoCorr_B]
  Register10/1 -> [regPktDet_pktDetMinDuration_autoCorr_C]
  Register11/1 -> [regPktDet_pktDetMinDuration_autoCorr_D]

== system wlan_phy_rx_pmd/Registers/Fanout1 ==
  In1 : Inport
  In2 : Inport  Port=2
  In3 : Inport  Port=3
  Register{1..11,20} (12 alike) : xbsIndex_r4/Register  init=0  rst=off  en=off  dbl_ovrd=off  block_type=register
  tag scope: regRx_PKTDET_RSSI_MIN_DUR_A=global, regRx_PKTDET_RSSI_MIN_DUR_B=global, regRx_PKTDET_RSSI_MIN_DUR_C=global, regRx_PKTDET_RSSI_MIN_DUR_D=global, regRx_PKTDET_RSSI_THRESH_A=global, regRx_PKTDET_RSSI_THRESH_B=global, regRx_PKTDET_RSSI_THRESH_C=global, regRx_PKTDET_RSSI_THRESH_D=global, regRx_RSSI_SUM_LEN_A=global, regRx_RSSI_SUM_LEN_B=global, regRx_RSSI_SUM_LEN_C=global, regRx_RSSI_SUM_LEN_D=global
  wires:
  Register3/1 -> [regRx_RSSI_SUM_LEN_A]
  Register2/1 -> [regRx_RSSI_SUM_LEN_B]
  Register1/1 -> [regRx_RSSI_SUM_LEN_C]
  Register20/1 -> [regRx_RSSI_SUM_LEN_D]
  In1/1 -> Register3/1, Register2/1, Register1/1, Register20/1
  In2/1 -> Register6/1, Register4/1, Register5/1, Register7/1
  In3/1 -> Register11/1, Register9/1, Register8/1, Register10/1
  Register11/1 -> [regRx_PKTDET_RSSI_MIN_DUR_A]
  Register9/1 -> [regRx_PKTDET_RSSI_MIN_DUR_B]
  Register8/1 -> [regRx_PKTDET_RSSI_MIN_DUR_C]
  Register10/1 -> [regRx_PKTDET_RSSI_MIN_DUR_D]
  Register7/1 -> [regRx_PKTDET_RSSI_THRESH_A]
  Register5/1 -> [regRx_PKTDET_RSSI_THRESH_B]
  Register4/1 -> [regRx_PKTDET_RSSI_THRESH_C]
  Register6/1 -> [regRx_PKTDET_RSSI_THRESH_D]

== system wlan_phy_rx_pmd/Registers/Fanout2 ==
  Corr_Thresh : Inport
  E_Thresh : Inport  Port=2
  Register{1..7,20} (8 alike) : xbsIndex_r4/Register  init=0  rst=off  en=off  dbl_ovrd=off  block_type=register
  tag scope: regRx_PktDet_CorrThresh_DSSS_A=global, regRx_PktDet_CorrThresh_DSSS_B=global, regRx_PktDet_CorrThresh_DSSS_C=global, regRx_PktDet_CorrThresh_DSSS_D=global, regRx_PktDet_EnergyThresh_DSSS_A=global, regRx_PktDet_EnergyThresh_DSSS_B=global, regRx_PktDet_EnergyThresh_DSSS_C=global, regRx_PktDet_EnergyThresh_DSSS_D=global
  wires:
  Register3/1 -> [regRx_PktDet_CorrThresh_DSSS_A]
  Register2/1 -> [regRx_PktDet_CorrThresh_DSSS_B]
  Register1/1 -> [regRx_PktDet_CorrThresh_DSSS_C]
  Register20/1 -> [regRx_PktDet_CorrThresh_DSSS_D]
  Corr_Thresh/1 -> Register3/1, Register2/1, Register1/1, Register20/1
  E_Thresh/1 -> Register4/1, Register5/1, Register6/1, Register7/1
  Register4/1 -> [regRx_PktDet_EnergyThresh_DSSS_A]
  Register5/1 -> [regRx_PktDet_EnergyThresh_DSSS_B]
  Register6/1 -> [regRx_PktDet_EnergyThresh_DSSS_C]
  Register7/1 -> [regRx_PktDet_EnergyThresh_DSSS_D]

== system wlan_phy_rx_pmd/Registers/Sim Reset ==
  Add : Sum
  Disregard Subsystem : xbsIndex_r4/Disregard Subsystem  Tag=discardX  block_type=disregard
  Pulse Generator : DiscretePulseGenerator  Period=1e6  PulseWidth=2  PhaseDelay=1.25e4
  Pulse Generator1 : DiscretePulseGenerator  Period=1e6  PulseWidth=2  PhaseDelay=2.5e4
  sim_only_reset : xbsIndex_r4/Gateway In  gui_display_data_type=Fixed-point  arith_type=Unsigned  n_bits=1  bin_pt=0  preci_type=Single  exp_width=8  frac_width=24  quantization="Round  (unbiased: +/- Inf)"  overflow=Saturate  period=1  dbl_ovrd=off  timing_constraint=None  locs_specified=off  LOCs={}  UseAsADC=off  ADCChannel='1'  inherit_from_input=off  hdl_port=on  block_type=gatewayin
  Out1 : Outport
  wires:
  sim_only_reset/1 -> Out1/1
  Pulse Generator/1 -> Add/1
  Pulse Generator1/1 -> Add/2
  Add/1 -> sim_only_reset/1
